simulink library "inp"  (Simulink 8.2)
settings: Solver=ode45  SolverMode=SingleTasking  StartTime=0.0  StopTime=10.0  RelTol=1e-3
undefined symbols (not defined in the file or in its folder): C_TICK_TIME_S, ENUM_FAULTSTATE_FAIL, ENUM_FAULTSTATE_INDETERMINATE, ENUM_FAULTSTATE_PASS, ENUM_KEYSWITCH_ON, FALSE, KINP_ABS1CANCommuOk_flg_ovrdflg, KINP_ABS1CANCommuOk_flg_ovrdval, KINP_ABS1CANPeriodTime_s, KINP_ABS1CANRolCntOk_flg_ovrdflg, KINP_ABS1CANRolCntOk_flg_ovrdval, KINP_ABS2CANChkSumOk_flg_ovrdflg, KINP_ABS2CANChkSumOk_flg_ovrdval, KINP_ABS2CANCommuOk_flg_ovrdflg, KINP_ABS2CANCommuOk_flg_ovrdval, KINP_ABS2CANPeriodTime_s, KINP_ABS2CANRolCntOk_flg_ovrdflg, KINP_ABS2CANRolCntOk_flg_ovrdval, KINP_ABS3CANCommuOk_flg_ovrdflg, KINP_ABS3CANCommuOk_flg_ovrdval, KINP_ABS4CANCommuOk_flg_ovrdflg, KINP_ABS4CANCommuOk_flg_ovrdval, KINP_ABSActive_flg_defval, KINP_ABSActive_flg_invalid, KINP_ABSActive_flg_ovrdflg, KINP_ABSActive_flg_ovrdval, KINP_ABSActive_flg_setvalid, KINP_ABSActive_flg_srvcflg, KINP_ABSActive_flg_srvcval, KINP_ABSCANAlive_flg_ovrdflg, KINP_ABSCANAlive_flg_ovrdval, KINP_ABSCANCommuOk_flg_ovrdflg, KINP_ABSCANCommuOk_flg_ovrdval, KINP_ABSCANValidOk_flg_ovrdflg, KINP_ABSCANValidOk_flg_ovrdval, KINP_ABSFault_flg_defval, KINP_ABSFault_flg_invalid, KINP_ABSFault_flg_ovrdflg, KINP_ABSFault_flg_ovrdval, KINP_ABSFault_flg_setvalid, KINP_ABSFault_flg_srvcflg, KINP_ABSFault_flg_srvcval, KINP_AC1CANCommuOk_flg_ovrdflg, KINP_AC1CANCommuOk_flg_ovrdval, KINP_ACCANAlive_flg_ovrdflg, KINP_ACCANAlive_flg_ovrdval, KINP_ACCANCommuOk_flg_ovrdflg, KINP_ACCANCommuOk_flg_ovrdval, KINP_ACCANValidOk_flg_ovrdflg, KINP_ACCANValidOk_flg_ovrdval, KINP_ACCCancelH_flg_defval, KINP_ACCCancelH_flg_invalid, KINP_ACCCancelH_flg_ovrdflg, KINP_ACCCancelH_flg_ovrdval, KINP_ACCCancelH_flg_setvalid, KINP_ACCCancelH_flg_srvcflg, KINP_ACCCancelH_flg_srvcval, KINP_AccelPedalPosn_pct_defval, KINP_AccelPedalPosn_pct_gain, KINP_AccelPedalPosn_pct_invalid ... (+1866)
libraries: inp x192, hcu_lib x34, simulink x33
(part 15 of 21)

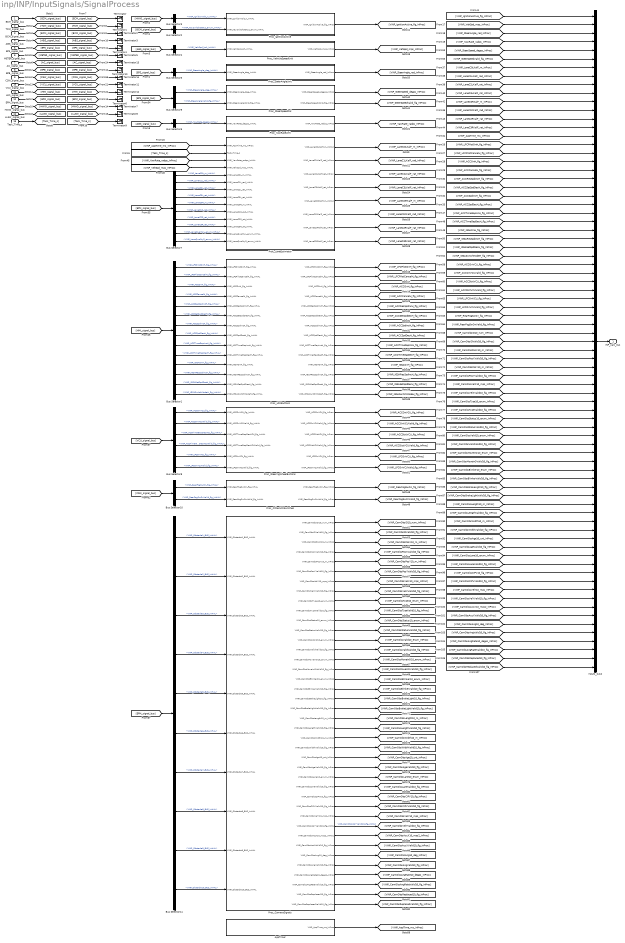
== system inp/INP/InputSignals/SignalProcess ==
  ECM_signal_bus : Inport
  TCM_signal_bus : Inport  Port=2
  BCM_signal_bus : Inport  Port=3
  ABS_signal_bus : Inport  Port=4
  EPS_signal_bus : Inport  Port=5
  METER_signal_bus : Inport  Port=6
  AC_signal_bus : Inport  Port=7
  EPB_signal_bus : Inport  Port=8
  CSW_signal_bus : Inport  Port=9
  VCU_signal_bus : Inport  Port=10
  HMI_signal_bus : Inport  Port=11
  EPM_signal_bus : Inport  Port=12
  HWIO_signal_bus : Inport  Port=13
  LLSW_signal_bus : Inport  Port=14
  Task_Time_s : Inport  Port=15
  AppTimer : Subsystem
  Bus Selector1 : BusSelector  OutputSignals=VINP_LFCPilotOnH_flg_InHAL,VINP_LFCPilotCancelH_flg_InHAL,VINP_ACCOnH_flg_InHAL,VINP_ACCCancelH_flg_InHAL,VINP_ACCResSpdIncH_flg_InHAL,VINP_ACCSetSpdDecH_flg_InHAL,VINP_ACCSpdIncH_flg_InHAL,VINP_ACCSpdDecH_flg_InHAL,VINP_ACCTimeGapIncH_flg_InHAL,VINP_ACCTimeGapDecH_flg_InHAL,VINP_IGSAOnH_flg_InHAL,VINP_IGSAResSpdIncH_flg_InHAL,VINP_IGSASetSpdDecH_flg_InHAL,VINP_IGSASwitchModeH_flg_InHAL
  Bus Selector10 : BusSelector  OutputSignals=VINP_RearFogSwOn_flg_InHAL,VINP_RearFogSwOnValid_flg_InHAL
  Bus Selector11 : BusSelector  OutputSignals=VINP_Obstacle1_BUS_InHAL,VINP_Obstacle2_BUS_InHAL,VINP_Obstacle3_BUS_InHAL,VINP_Obstacle4_BUS_InHAL,VINP_Obstacle5_BUS_InHAL,VINP_Obstacle6_BUS_InHAL,VINP_Obstacle7_BUS_InHAL,VINP_Obstacle8_BUS_InHAL,VINP_Obstacle9_BUS_InHAL,VINP_Obstacle10_BUS_InHAL
  Bus Selector2 : BusSelector  OutputSignals=VINP_KeySwitchStatus_enum_InHAL
  Bus Selector3 : BusSelector  OutputSignals=VINP_IgnitionVolt_V_InHAL
  Bus Selector4 : BusSelector  OutputSignals=VINP_VehSpd_kph_InHAL
  Bus Selector5 : BusSelector  OutputSignals=VINP_SteerAngle_deg_InHAL
  Bus Selector6 : BusSelector  OutputSignals=VINP_YawRate_degps_InHAL
  Bus Selector7 : BusSelector  OutputSignals=VINP_LaneC0L_m_InHAL,VINP_LaneC1L_rad_InHAL,VINP_LaneC2L_rat_InHAL,VINP_LaneC3L_rat_InHAL,VINP_LaneC0R_m_InHAL,VINP_LaneC1R_rad_InHAL,VINP_LaneC2R_rat_InHAL,VINP_LaneC3R_rat_InHAL,VINP_LaneQualityL_enum_InHAL,VINP_LaneQualityR_enum_InHAL
  Bus Selector8 : BusSelector  OutputSignals=VINP_ACCOnVCU_flg_InHAL,VINP_ACCOnVCUValid_flg_InHAL,VINP_ACCTimeGapDecVCU_flg_InHAL,VINP_ACCTimeGapDecVCUValid_flg_InHAL,VINP_LFCOnVCU_flg_InHAL,VINP_LFCOnVCUValid_flg_InHAL
  Bus Selector9 : BusSelector  OutputSignals=VINP_SteerAngle_deg_InHAL,VINP_SteerAngleValid_flg_InHAL
  Proc_CameraSignals : Subsystem
  Proc_HMIButtons : Subsystem
  Proc_IgnitionActive : Subsystem
  Proc_LaneEstimator : Subsystem
  Proc_OtherHMISwitches : Subsystem
  Proc_SteerAngleUnit : Subsystem
  Proc_SteerSpeedCal : Subsystem
  Proc_SteeringWheelButtons : Subsystem
  Proc_VehicleSpeedUnit : Subsystem
  Proc_YawRateUnit : Subsystem
  Terminator{,1..14} (15 alike) : Terminator
  inputs_bus2 : BusCreator  Inputs=77
  INP_calc_bus : Outport
  wires:
  [VINP_YawRate_radps_InProc] -> inputs_bus2/4
  [VINP_SteerAngle_rad_InProc] -> inputs_bus2/3
  [VINP_VehSpd_mps_InProc] -> inputs_bus2/2
  [VINP_IgnitionActive_flg_InProc] -> inputs_bus2/1
  AppTimer/1(VINP_AppTime_ms_InProc) -> [VINP_AppTime_ms_InProc]
  LLSW_signal_bus/1 -> [LLSW_signal_bus]
  Task_Time_s/1 -> [Task_Time_s]
  HWIO_signal_bus/1 -> [HWIO_signal_bus]
  EPM_signal_bus/1 -> [EPM_signal_bus]
  EPS_signal_bus/1 -> [EPS_signal_bus]
  ABS_signal_bus/1 -> [ABS_signal_bus]
  BCM_signal_bus/1 -> [BCM_signal_bus]
  TCM_signal_bus/1 -> [TCM_signal_bus]
  ECM_signal_bus/1 -> [ECM_signal_bus]
  [BCM_signal_bus] -> Bus Selector2/1
  [HWIO_signal_bus] -> Bus Selector3/1
  Bus Selector3/1 -> Proc_IgnitionActive/1(VINP_IgnitionVolt_V_InHAL)  "<VINP_IgnitionVolt_V_InHAL>"
  Bus Selector2/1 -> Proc_IgnitionActive/2(VINP_KeySwitchStatus_enum_InHAL)  "<VINP_KeySwitchStatus_enum_InHAL>"
  Proc_IgnitionActive/1(VINP_IgnitionActive_flg_InProc) -> [VINP_IgnitionActive_flg_InProc]
  [ABS_signal_bus] -> Bus Selector4/1
  Bus Selector4/1 -> Proc_VehicleSpeedUnit/1(VINP_VehSpd_kph_InHAL)  "<VINP_VehSpd_kph_InHAL>"
  Proc_VehicleSpeedUnit/1(VINP_VehSpd_mps_InProc) -> [VINP_VehSpd_mps_InProc]
  [EPS_signal_bus] -> Bus Selector5/1
  Bus Selector5/1 -> Proc_SteerAngleUnit/1(VINP_SteerAngle_deg_InHAL)  "<VINP_SteerAngle_deg_InHAL>"
  [ABS_signal_bus] -> Bus Selector6/1
  Bus Selector6/1 -> Proc_YawRateUnit/1(VINP_YawRate_degps_InHAL)  "<VINP_YawRate_degps_InHAL>"
  Proc_SteerAngleUnit/1(VINP_SteerAngle_rad_InProc) -> [VINP_SteerAngle_rad_InProc]
  Proc_YawRateUnit/1(VINP_YawRate_radps_InProc) -> [VINP_YawRate_radps_InProc]
  [ECM_signal_bus] -> Terminator/1
  [TCM_signal_bus] -> Terminator1/1
  [BCM_signal_bus] -> Terminator2/1
  [ABS_signal_bus] -> Terminator3/1
  [EPS_signal_bus] -> Terminator4/1
  [EPM_signal_bus] -> Terminator6/1
  [HWIO_signal_bus] -> Terminator7/1
  [LLSW_signal_bus] -> Terminator8/1
  [Task_Time_s] -> Terminator9/1
  inputs_bus2/1 -> INP_calc_bus/1
  [VINP_AppTime_ms_InProc] -> inputs_bus2/15
  [VINP_LaneC0LKalF_m_InProc] -> inputs_bus2/7
  [VINP_LaneC1LKalF_rad_InProc] -> inputs_bus2/8
  [VINP_LaneC2LKalF_rat_InProc] -> inputs_bus2/9
  [VINP_LaneC3LKalF_rat_InProc] -> inputs_bus2/10
  [VINP_LaneC0RKalF_m_InProc] -> inputs_bus2/11
  [VINP_LaneC1RKalF_rad_InProc] -> inputs_bus2/12
  [VINP_LaneC2RKalF_rat_InProc] -> inputs_bus2/13
  [VINP_LaneC3RKalF_rat_InProc] -> inputs_bus2/14
  [EPM_signal_bus] -> Bus Selector7/1
  Bus Selector7/1 -> Proc_LaneEstimator/5(VINP_LaneC0L_m_InHAL)  "<VINP_LaneC0L_m_InHAL>"
  Bus Selector7/2 -> Proc_LaneEstimator/6(VINP_LaneC1L_rad_InHAL)  "<VINP_LaneC1L_rad_InHAL>"
  Bus Selector7/3 -> Proc_LaneEstimator/7(VINP_LaneC2L_rat_InHAL)  "<VINP_LaneC2L_rat_InHAL>"
  Bus Selector7/4 -> Proc_LaneEstimator/8(VINP_LaneC3L_rat_InHAL)  "<VINP_LaneC3L_rat_InHAL>"
  Bus Selector7/5 -> Proc_LaneEstimator/9(VINP_LaneC0R_m_InHAL)  "<VINP_LaneC0R_m_InHAL>"
  Bus Selector7/6 -> Proc_LaneEstimator/10(VINP_LaneC1R_rad_InHAL)  "<VINP_LaneC1R_rad_InHAL>"
  Bus Selector7/7 -> Proc_LaneEstimator/11(VINP_LaneC2R_rat_InHAL)  "<VINP_LaneC2R_rat_InHAL>"
  Bus Selector7/8 -> Proc_LaneEstimator/12(VINP_LaneC3R_rat_InHAL)  "<VINP_LaneC3R_rat_InHAL>"
  [VINP_YawRate_radps_InProc] -> Proc_LaneEstimator/3(VINP_YawRate_radps_InHAL)
  [VINP_VehSpd_mps_InProc] -> Proc_LaneEstimator/4(VINP_VehSpd_mps_InHAL)
  Bus Selector7/9 -> Proc_LaneEstimator/13(VINP_LaneQualityL_enum_InHAL)  "<VINP_LaneQualityL_enum_InHAL>"
  Bus Selector7/10 -> Proc_LaneEstimator/14(VINP_LaneQualityR_enum_InHAL)  "<VINP_LaneQualityR_enum_InHAL>"
  [Task_Time_s] -> Proc_LaneEstimator/2(Task_Time_s)
  [VINP_AppTime_ms_InProc] -> Proc_LaneEstimator/1(VINP_AppTime_ms_InProc)
  Proc_LaneEstimator/1(VINP_LaneC0LKalF_m_InProc) -> [VINP_LaneC0LKalF_m_InProc]
  Proc_LaneEstimator/2(VINP_LaneC1LKalF_rad_InProc) -> [VINP_LaneC1LKalF_rad_InProc]
  Proc_LaneEstimator/3(VINP_LaneC2LKalF_rat_InProc) -> [VINP_LaneC2LKalF_rat_InProc]
  Proc_LaneEstimator/4(VINP_LaneC3LKalF_rat_InProc) -> [VINP_LaneC3LKalF_rat_InProc]
  Proc_LaneEstimator/5(VINP_LaneC0RKalF_m_InProc) -> [VINP_LaneC0RKalF_m_InProc]
  Proc_LaneEstimator/6(VINP_LaneC1RKalF_rad_InProc) -> [VINP_LaneC1RKalF_rad_InProc]
  Proc_LaneEstimator/7(VINP_LaneC2RKalF_rat_InProc) -> [VINP_LaneC2RKalF_rat_InProc]
  Proc_LaneEstimator/8(VINP_LaneC3RKalF_rat_InProc) -> [VINP_LaneC3RKalF_rat_InProc]
  [METER_signal_bus] -> Terminator5/1
  METER_signal_bus/1 -> [METER_signal_bus]
  [AC_signal_bus] -> Terminator13/1
  AC_signal_bus/1 -> [AC_signal_bus]
  [EPB_signal_bus] -> Terminator10/1
  EPB_signal_bus/1 -> [EPB_signal_bus]
  [VCU_signal_bus] -> Terminator11/1
  VCU_signal_bus/1 -> [VCU_signal_bus]
  [HMI_signal_bus] -> Terminator12/1
  HMI_signal_bus/1 -> [HMI_signal_bus]
  [HMI_signal_bus] -> Bus Selector1/1
  Bus Selector1/1 -> Proc_HMIButtons/1(VINP_LFCPilotOnH_flg_InHAL)  "<VINP_LFCPilotOnH_flg_InHAL>"
  Proc_HMIButtons/1(VINP_LFCPilotOnH_flg_InProc) -> [VINP_LFCPilotOnH_flg_InProc]
  Bus Selector1/2 -> Proc_HMIButtons/2(VINP_LFCPilotCancelH_flg_InHAL)  "<VINP_LFCPilotCancelH_flg_InHAL>"
  Bus Selector1/3 -> Proc_HMIButtons/3(VINP_ACCOnH_flg_InHAL)  "<VINP_ACCOnH_flg_InHAL>"
  Bus Selector1/4 -> Proc_HMIButtons/4(VINP_ACCCancelH_flg_InHAL)  "<VINP_ACCCancelH_flg_InHAL>"
  Bus Selector1/5 -> Proc_HMIButtons/5(VINP_ACCResSpdIncH_flg_InHAL)  "<VINP_ACCResSpdIncH_flg_InHAL>"
  Bus Selector1/6 -> Proc_HMIButtons/6(VINP_ACCSetSpdDecH_flg_InHAL)  "<VINP_ACCSetSpdDecH_flg_InHAL>"
  Bus Selector1/7 -> Proc_HMIButtons/7(VINP_ACCSpdIncH_flg_InHAL)  "<VINP_ACCSpdIncH_flg_InHAL>"
  Bus Selector1/8 -> Proc_HMIButtons/8(VINP_ACCSpdDecH_flg_InHAL)  "<VINP_ACCSpdDecH_flg_InHAL>"
  Bus Selector1/9 -> Proc_HMIButtons/9(VINP_ACCTimeGapIncH_flg_InHAL)  "<VINP_ACCTimeGapIncH_flg_InHAL>"
  Bus Selector1/10 -> Proc_HMIButtons/10(VINP_ACCTimeGapDecH_flg_InHAL)  "<VINP_ACCTimeGapDecH_flg_InHAL>"
  Bus Selector1/11 -> Proc_HMIButtons/11(VINP_IGSAOnH_flg_InHAL)  "<VINP_IGSAOnH_flg_InHAL>"
  Bus Selector1/12 -> Proc_HMIButtons/12(VINP_IGSAResSpdIncH_flg_InHAL)  "<VINP_IGSAResSpdIncH_flg_InHAL>"
  Bus Selector1/13 -> Proc_HMIButtons/13(VINP_IGSASetSpdDecH_flg_InHAL)  "<VINP_IGSASetSpdDecH_flg_InHAL>"
  Bus Selector1/14 -> Proc_HMIButtons/14(VINP_IGSASwitchModeH_flg_InHAL)  "<VINP_IGSASwitchModeH_flg_InHAL>"
  Proc_HMIButtons/2(VINP_LFCPilotCancelH_flg_InProc) -> [VINP_LFCPilotCancelH_flg_InProc]
  Proc_HMIButtons/3(VINP_ACCOnH_flg_InProc) -> [VINP_ACCOnH_flg_InProc]
  Proc_HMIButtons/4(VINP_ACCCancelH_flg_InProc) -> [VINP_ACCCancelH_flg_InProc]
  Proc_HMIButtons/5(VINP_ACCResSpdIncH_flg_InProc) -> [VINP_ACCResSpdIncH_flg_InProc]
  Proc_HMIButtons/6(VINP_ACCSetSpdDecH_flg_InProc) -> [VINP_ACCSetSpdDecH_flg_InProc]
  Proc_HMIButtons/7(VINP_ACCSpdIncH_flg_InProc) -> [VINP_ACCSpdIncH_flg_InProc]
  Proc_HMIButtons/8(VINP_ACCSpdDecH_flg_InProc) -> [VINP_ACCSpdDecH_flg_InProc]
  Proc_HMIButtons/9(VINP_ACCTimeGapIncH_flg_InProc) -> [VINP_ACCTimeGapIncH_flg_InProc]
  Proc_HMIButtons/10(VINP_ACCTimeGapDecH_flg_InProc) -> [VINP_ACCTimeGapDecH_flg_InProc]
  Proc_HMIButtons/11(VINP_IGSAOnH_flg_InProc) -> [VINP_IGSAOnH_flg_InProc]
  Proc_HMIButtons/12(VINP_IGSAResSpdIncH_flg_InProc) -> [VINP_IGSAResSpdIncH_flg_InProc]
  Proc_HMIButtons/13(VINP_IGSASetSpdDecH_flg_InProc) -> [VINP_IGSASetSpdDecH_flg_InProc]
  Proc_HMIButtons/14(VINP_IGSASwitchModeH_flg_InProc) -> [VINP_IGSASwitchModeH_flg_InProc]
  [VINP_LFCPilotOnH_flg_InProc] -> inputs_bus2/16
  [VINP_LFCPilotCancelH_flg_InProc] -> inputs_bus2/17
  [VINP_ACCOnH_flg_InProc] -> inputs_bus2/18
  [VINP_ACCCancelH_flg_InProc] -> inputs_bus2/19
  [VINP_ACCResSpdIncH_flg_InProc] -> inputs_bus2/20
  [VINP_ACCSetSpdDecH_flg_InProc] -> inputs_bus2/21
  [VINP_ACCSpdIncH_flg_InProc] -> inputs_bus2/22
  [VINP_ACCSpdDecH_flg_InProc] -> inputs_bus2/23
  [VINP_ACCTimeGapIncH_flg_InProc] -> inputs_bus2/24
  [VINP_ACCTimeGapDecH_flg_InProc] -> inputs_bus2/25
  [VINP_IGSAOnH_flg_InProc] -> inputs_bus2/26
  [VINP_IGSAResSpdIncH_flg_InProc] -> inputs_bus2/27
  [VINP_IGSASetSpdDecH_flg_InProc] -> inputs_bus2/28
  [VINP_IGSASwitchModeH_flg_InProc] -> inputs_bus2/29
  Proc_SteerSpeedCal/1(VINP_SteerSpeed_degps_InProc) -> [VINP_SteerSpeed_degps_InProc]
  [EPS_signal_bus] -> Bus Selector9/1
  Bus Selector9/1 -> Proc_SteerSpeedCal/1(VINP_SteerAngle_deg_InHAL)  "<VINP_SteerAngle_deg_InHAL>"
  Bus Selector9/2 -> Proc_SteerSpeedCal/2(VINP_SteerAngleValid_flg_InHAL)  "<VINP_SteerAngleValid_flg_InHAL>"
  Proc_SteerSpeedCal/2(VINP_SteerSpeedValid_flg_InProc) -> [VINP_SteerSpeedValid_flg_InProc]
  [VINP_SteerSpeed_degps_InProc] -> inputs_bus2/5
  [VINP_SteerSpeedValid_flg_InProc] -> inputs_bus2/6
  [CSW_signal_bus] -> Terminator14/1
  CSW_signal_bus/1 -> [CSW_signal_bus]
  [VCU_signal_bus] -> Bus Selector8/1
  Bus Selector8/2 -> Proc_SteeringWheelButtons/2(VINP_ACCOnVCUValid_flg_InHAL)  "<VINP_ACCOnVCUValid_flg_InHAL>"
  Bus Selector8/3 -> Proc_SteeringWheelButtons/3(VINP_ACCTimeGapDecVCU_flg_InHAL)  "<VINP_ACCTimeGapDecVCU_flg_InHAL>"
  Bus Selector8/1 -> Proc_SteeringWheelButtons/1(VINP_ACCOnVCU_flg_InHAL)  "<VINP_ACCOnVCU_flg_InHAL>"
  Bus Selector8/4 -> Proc_SteeringWheelButtons/4(VINP_ACCTimeGapDecVCUValid_flg_InHAL)  "<VINP_ACCTimeGapDecVCUValid_flg_InHAL>"
  Proc_SteeringWheelButtons/1(VINP_ACCOnVCU_flg_InProc) -> [VINP_ACCOnVCU_flg_InProc]
  Proc_SteeringWheelButtons/2(VINP_ACCOnVCUValid_flg_InProc) -> [VINP_ACCOnVCUValid_flg_InProc]
  Proc_SteeringWheelButtons/3(VINP_ACCDistVCU_flg_InProc) -> [VINP_ACCDistVCU_flg_InProc]
  Proc_SteeringWheelButtons/4(VINP_ACCDistVCUValid_flg_InProc) -> [VINP_ACCDistVCUValid_flg_InProc]
  [VINP_ACCOnVCU_flg_InProc] -> inputs_bus2/30
  [VINP_ACCOnVCUValid_flg_InProc] -> inputs_bus2/31
  [VINP_ACCDistVCU_flg_InProc] -> inputs_bus2/32
  [VINP_ACCDistVCUValid_flg_InProc] -> inputs_bus2/33
  [CSW_signal_bus] -> Bus Selector10/1
  Bus Selector10/1 -> Proc_OtherHMISwitches/1(VINP_RearFogSwOn_flg_InHAL)  "<VINP_RearFogSwOn_flg_InHAL>"
  Proc_OtherHMISwitches/1(VINP_RearFogSwOn_flg_InProc) -> [VINP_RearFogSwOn_flg_InProc]
  [VINP_RearFogSwOn_flg_InProc] -> inputs_bus2/36
  Bus Selector10/2 -> Proc_OtherHMISwitches/2(VINP_RearFogSwOnValid_flg_InHAL)  "<VINP_RearFogSwOnValid_flg_InHAL>"
  Proc_OtherHMISwitches/2(VINP_RearFogSwOnValid_flg_InProc) -> [VINP_RearFogSwOnValid_flg_InProc]
  [VINP_RearFogSwOnValid_flg_InProc] -> inputs_bus2/37
  Bus Selector8/5 -> Proc_SteeringWheelButtons/5(VINP_LFCOnVCU_flg_InHAL)  "<VINP_LFCOnVCU_flg_InHAL>"
  Bus Selector8/6 -> Proc_SteeringWheelButtons/6(VINP_LFCOnVCUValid_flg_InHAL)  "<VINP_LFCOnVCUValid_flg_InHAL>"
  Proc_SteeringWheelButtons/5(VINP_LFCOnVCU_flg_InProc) -> [VINP_LFCOnVCU_flg_InProc]
  Proc_SteeringWheelButtons/6(VINP_LFCOnVCUValid_flg_InProc) -> [VINP_LFCOnVCUValid_flg_InProc]
  [VINP_LFCOnVCU_flg_InProc] -> inputs_bus2/34
  [VINP_LFCOnVCUValid_flg_InProc] -> inputs_bus2/35
  [EPM_signal_bus] -> Bus Selector11/1
  Bus Selector11/1 -> Proc_CameraSignals/1(VINP_Obstacle1_BUS_InHAL)  "<VINP_Obstacle1_BUS_InHAL>"
  Bus Selector11/2 -> Proc_CameraSignals/2(VINP_Obstacle2_BUS_InHAL)  "<VINP_Obstacle2_BUS_InHAL>"
  Bus Selector11/3 -> Proc_CameraSignals/3(VINP_Obstacle3_BUS_InHAL)  "<VINP_Obstacle3_BUS_InHAL>"
  Bus Selector11/4 -> Proc_CameraSignals/4(VINP_Obstacle4_BUS_InHAL)  "<VINP_Obstacle4_BUS_InHAL>"
  Bus Selector11/5 -> Proc_CameraSignals/5(VINP_Obstacle5_BUS_InHAL)  "<VINP_Obstacle5_BUS_InHAL>"
  Bus Selector11/6 -> Proc_CameraSignals/6(VINP_Obstacle6_BUS_InHAL)  "<VINP_Obstacle6_BUS_InHAL>"
  Bus Selector11/7 -> Proc_CameraSignals/7(VINP_Obstacle7_BUS_InHAL)  "<VINP_Obstacle7_BUS_InHAL>"
  Bus Selector11/8 -> Proc_CameraSignals/8(VINP_Obstacle8_BUS_InHAL)  "<VINP_Obstacle8_BUS_InHAL>"
  Bus Selector11/9 -> Proc_CameraSignals/9(VINP_Obstacle9_BUS_InHAL)  "<VINP_Obstacle9_BUS_InHAL>"
  Bus Selector11/10 -> Proc_CameraSignals/10(VINP_Obstacle10_BUS_InHAL)  "<VINP_Obstacle10_BUS_InHAL>"
  Proc_CameraSignals/1(VINP_CamObsID10_num_InProc) -> [VINP_CamObsID10_num_InProc]
  Proc_CameraSignals/2(VINP_CamObsIDValid10_flg_InProc) -> [VINP_CamObsIDValid10_flg_InProc]
  Proc_CameraSignals/3(VINP_CamObsPosX10_m_InProc) -> [VINP_CamObsPosX10_m_InProc]
  Proc_CameraSignals/4(VINP_CamObsPosXValid10_flg_InProc) -> [VINP_CamObsPosXValid10_flg_InProc]
  Proc_CameraSignals/5(VINP_CamObsPosY10_m_InProc) -> [VINP_CamObsPosY10_m_InProc]
  Proc_CameraSignals/6(VINP_CamObsPosYValid10_flg_InProc) -> [VINP_CamObsPosYValid10_flg_InProc]
  Proc_CameraSignals/7(VINP_CamObsVelX10_mps_InProc) -> [VINP_CamObsVelX10_mps_InProc]
  Proc_CameraSignals/8(VINP_CamObsVelXValid10_flg_InProc) -> [VINP_CamObsVelXValid10_flg_InProc]
  Proc_CameraSignals/9(VINP_CamObsType10_enum_InProc) -> [VINP_CamObsType10_enum_InProc]
  Proc_CameraSignals/10(VINP_CamObsTypeValid10_flg_InProc) -> [VINP_CamObsTypeValid10_flg_InProc]
  Proc_CameraSignals/11(VINP_CamObsStatus10_enum_InProc) -> [VINP_CamObsStatus10_enum_InProc]
  Proc_CameraSignals/12(VINP_CamObsStatusValid10_flg_InProc) -> [VINP_CamObsStatusValid10_flg_InProc]
  Proc_CameraSignals/13(VINP_CamObsValid10_enum_InProc) -> [VINP_CamObsValid10_enum_InProc]
  Proc_CameraSignals/14(VINP_CamObsValidValid10_flg_InProc) -> [VINP_CamObsValidValid10_flg_InProc]
  Proc_CameraSignals/15(VINP_CamObsMoveInO10_enum_InProc) -> [VINP_CamObsMoveInO10_enum_InProc]
  Proc_CameraSignals/16(VINP_CamObsMoveInOValid10_flg_InProc) -> [VINP_CamObsMoveInOValid10_flg_InProc]
  Proc_CameraSignals/17(VINP_CamObsBlinker10_enum_InProc) -> [VINP_CamObsBlinker10_enum_InProc]
  Proc_CameraSignals/18(VINP_CamObsBlinkerValid10_flg_InProc) -> [VINP_CamObsBlinkerValid10_flg_InProc]
  Proc_CameraSignals/19(VINP_CamObsBrakeLight10_flg_InProc) -> [VINP_CamObsBrakeLight10_flg_InProc]
  Proc_CameraSignals/20(VINP_CamObsBrakeLightValid10_flg_InProc) -> [VINP_CamObsBrakeLightValid10_flg_InProc]
  Proc_CameraSignals/21(VINP_CamObsLength10_m_InProc) -> [VINP_CamObsLength10_m_InProc]
  Proc_CameraSignals/22(VINP_CamObsLengthValid10_flg_InProc) -> [VINP_CamObsLengthValid10_flg_InProc]
  Proc_CameraSignals/23(VINP_CamObsWidth10_m_InProc) -> [VINP_CamObsWidth10_m_InProc]
  Proc_CameraSignals/24(VINP_CamObsWidthValid10_flg_InProc) -> [VINP_CamObsWidthValid10_flg_InProc]
  Proc_CameraSignals/25(VINP_CamObsAge10_cnt_InProc) -> [VINP_CamObsAge10_cnt_InProc]
  Proc_CameraSignals/26(VINP_CamObsAgeValid10_flg_InProc) -> [VINP_CamObsAgeValid10_flg_InProc]
  Proc_CameraSignals/27(VINP_CamObsLane10_enum_InProc) -> [VINP_CamObsLane10_enum_InProc]
  Proc_CameraSignals/28(VINP_CamObsLaneValid10_flg_InProc) -> [VINP_CamObsLaneValid10_flg_InProc]
  Proc_CameraSignals/29(VINP_CamObsCIPV10_flg_InProc) -> [VINP_CamObsCIPV10_flg_InProc]
  Proc_CameraSignals/30(VINP_CamObsCIPVValid10_flg_InProc) -> [VINP_CamObsCIPVValid10_flg_InProc]
  Proc_CameraSignals/31(VINP_CamObsVelY10_mps_InProc) -> [VINP_CamObsVelY10_mps_InProc]
  Proc_CameraSignals/32(VINP_CamObsVelYValid10_flg_InProc) -> [VINP_CamObsVelYValid10_flg_InProc]  "VINP_CamObsVelYValid10_flg_InProc"
  Proc_CameraSignals/33(VINP_CamObsAccX10_mps2_InProc) -> [VINP_CamObsAccX10_mps2_InProc]
  Proc_CameraSignals/34(VINP_CamObsAccXValid10_flg_InProc) -> [VINP_CamObsAccXValid10_flg_InProc]
  Proc_CameraSignals/35(VINP_CamObsAng10_deg_InProc) -> [VINP_CamObsAng10_deg_InProc]
  Proc_CameraSignals/36(VINP_CamObsAngValid10_flg_InProc) -> [VINP_CamObsAngValid10_flg_InProc]
  Proc_CameraSignals/37(VINP_CamObsAngRate10_degps_InProc) -> [VINP_CamObsAngRate10_degps_InProc]
  Proc_CameraSignals/38(VINP_CamObsAngRateValid10_flg_InProc) -> [VINP_CamObsAngRateValid10_flg_InProc]
  Proc_CameraSignals/39(VINP_CamObsReplaced10_flg_InProc) -> [VINP_CamObsReplaced10_flg_InProc]
  Proc_CameraSignals/40(VINP_CamObsReplacedValid10_flg_InProc) -> [VINP_CamObsReplacedValid10_flg_InProc]
  [VINP_CamObsID10_num_InProc] -> inputs_bus2/38
  [VINP_CamObsIDValid10_flg_InProc] -> inputs_bus2/39
  [VINP_CamObsPosX10_m_InProc] -> inputs_bus2/40
  [VINP_CamObsPosXValid10_flg_InProc] -> inputs_bus2/41
  [VINP_CamObsPosY10_m_InProc] -> inputs_bus2/42
  [VINP_CamObsPosYValid10_flg_InProc] -> inputs_bus2/43
  [VINP_CamObsVelX10_mps_InProc] -> inputs_bus2/44
  [VINP_CamObsVelXValid10_flg_InProc] -> inputs_bus2/45
  [VINP_CamObsType10_enum_InProc] -> inputs_bus2/46
  [VINP_CamObsTypeValid10_flg_InProc] -> inputs_bus2/47
  [VINP_CamObsStatus10_enum_InProc] -> inputs_bus2/48
  [VINP_CamObsStatusValid10_flg_InProc] -> inputs_bus2/49
  [VINP_CamObsValid10_enum_InProc] -> inputs_bus2/50
  [VINP_CamObsValidValid10_flg_InProc] -> inputs_bus2/51
  [VINP_CamObsMoveInO10_enum_InProc] -> inputs_bus2/52
  [VINP_CamObsMoveInOValid10_flg_InProc] -> inputs_bus2/53
  [VINP_CamObsBlinker10_enum_InProc] -> inputs_bus2/54
  [VINP_CamObsBlinkerValid10_flg_InProc] -> inputs_bus2/55
  [VINP_CamObsBrakeLight10_flg_InProc] -> inputs_bus2/56
  [VINP_CamObsBrakeLightValid10_flg_InProc] -> inputs_bus2/57
  [VINP_CamObsLength10_m_InProc] -> inputs_bus2/58
  [VINP_CamObsLengthValid10_flg_InProc] -> inputs_bus2/59
  [VINP_CamObsWidth10_m_InProc] -> inputs_bus2/60
  [VINP_CamObsWidthValid10_flg_InProc] -> inputs_bus2/61
  [VINP_CamObsAge10_cnt_InProc] -> inputs_bus2/62
  [VINP_CamObsAgeValid10_flg_InProc] -> inputs_bus2/63
  [VINP_CamObsLane10_enum_InProc] -> inputs_bus2/64
  [VINP_CamObsLaneValid10_flg_InProc] -> inputs_bus2/65
  [VINP_CamObsCIPV10_flg_InProc] -> inputs_bus2/66
  [VINP_CamObsCIPVValid10_flg_InProc] -> inputs_bus2/67
  [VINP_CamObsVelY10_mps_InProc] -> inputs_bus2/68
  [VINP_CamObsVelYValid10_flg_InProc] -> inputs_bus2/69
  [VINP_CamObsAccX10_mps2_InProc] -> inputs_bus2/70
  [VINP_CamObsAccXValid10_flg_InProc] -> inputs_bus2/71
  [VINP_CamObsAng10_deg_InProc] -> inputs_bus2/72
  [VINP_CamObsAngValid10_flg_InProc] -> inputs_bus2/73
  [VINP_CamObsAngRate10_degps_InProc] -> inputs_bus2/74
  [VINP_CamObsAngRateValid10_flg_InProc] -> inputs_bus2/75
  [VINP_CamObsReplaced10_flg_InProc] -> inputs_bus2/76
  [VINP_CamObsReplacedValid10_flg_InProc] -> inputs_bus2/77

== system inp/INP/METER_signals/LowBrakeFluid ==
  signal_in : Inport
  msg_valid : Inport  Port=2
  Active_fault_flg : Inport  Port=3
  can_signal_process_wio_validity : inp/can_signal_process_wio_validity
  defval : Constant  Value=KINP_LowBrakeFluid_flg_defval
  invalid : Constant  Value=KINP_LowBrakeFluid_flg_invalid
  ovrdflg : Constant  Value=KINP_LowBrakeFluid_flg_ovrdflg
  ovrdval : Constant  Value=KINP_LowBrakeFluid_flg_ovrdval
  setvalid : Constant  Value=KINP_LowBrakeFluid_flg_setvalid
  srvcflg : Constant  Value=KINP_LowBrakeFluid_flg_srvcflg
  srvcval : Constant  Value=KINP_LowBrakeFluid_flg_srvcval
  signal_out : Outport
  signal_valid_out : Outport  Port=2
  wires:
  can_signal_process_wio_validity/1 -> signal_out/1
  can_signal_process_wio_validity/2 -> signal_valid_out/1
  signal_in/1 -> can_signal_process_wio_validity/1
  msg_valid/1 -> can_signal_process_wio_validity/2
  defval/1 -> can_signal_process_wio_validity/4
  invalid/1 -> can_signal_process_wio_validity/5
  setvalid/1 -> can_signal_process_wio_validity/6
  ovrdflg/1 -> can_signal_process_wio_validity/7
  ovrdval/1 -> can_signal_process_wio_validity/8
  srvcflg/1 -> can_signal_process_wio_validity/9
  srvcval/1 -> can_signal_process_wio_validity/10
  Active_fault_flg/1 -> can_signal_process_wio_validity/3

== system inp/INP/METER_signals/MeterVehSpd ==
  signal_in : Inport
  signal_valid_in : Inport  Port=2
  msg_valid : Inport  Port=3
  Active_fault_flg : Inport  Port=4
  can_signal_process_with_validity : inp/can_signal_process_with_validity
  conv_gain_offset : Subsystem
  defval : Constant  Value=KINP_MeterVehSpd_kph_defval
  invalid : Constant  Value=KINP_MeterVehSpd_kph_invalid
  min_max_limit : Subsystem
  ovrdflg : Constant  Value=KINP_MeterVehSpd_kph_ovrdflg
  ovrdval : Constant  Value=KINP_MeterVehSpd_kph_ovrdval
  setvalid : Constant  Value=KINP_MeterVehSpd_kph_setvalid
  srvcflg : Constant  Value=KINP_MeterVehSpd_kph_srvcflg
  srvcval : Constant  Value=KINP_MeterVehSpd_kph_srvcval
  signal_out : Outport
  signal_valid_out : Outport  Port=2
  raw_signal_valid_out : Outport  Port=3
  wires:
  can_signal_process_with_validity/1 -> min_max_limit/1(in)
  can_signal_process_with_validity/2 -> signal_valid_out/1
  signal_in/1 -> conv_gain_offset/1(in)
  signal_valid_in/1 -> can_signal_process_with_validity/2
  msg_valid/1 -> can_signal_process_with_validity/3, can_signal_process_with_validity/5
  defval/1 -> can_signal_process_with_validity/6
  invalid/1 -> can_signal_process_with_validity/7
  conv_gain_offset/1(out) -> can_signal_process_with_validity/1
  min_max_limit/1(out) -> signal_out/1
  setvalid/1 -> can_signal_process_with_validity/8
  ovrdflg/1 -> can_signal_process_with_validity/9
  ovrdval/1 -> can_signal_process_with_validity/10
  srvcflg/1 -> can_signal_process_with_validity/11
  srvcval/1 -> can_signal_process_with_validity/12
  Active_fault_flg/1 -> can_signal_process_with_validity/4
  can_signal_process_with_validity/3 -> raw_signal_valid_out/1

== system inp/INP/METER_signals/OdoMeter ==
  signal_in : Inport
  signal_valid_in : Inport  Port=2
  msg_valid : Inport  Port=3
  Active_fault_flg : Inport  Port=4
  can_signal_process_with_validity : inp/can_signal_process_with_validity
  conv_gain_offset : Subsystem
  defval : Constant  Value=KINP_OdoMeter_km_defval
  invalid : Constant  Value=KINP_OdoMeter_km_invalid
  min_max_limit : Subsystem
  ovrdflg : Constant  Value=KINP_OdoMeter_km_ovrdflg
  ovrdval : Constant  Value=KINP_OdoMeter_km_ovrdval
  setvalid : Constant  Value=KINP_OdoMeter_km_setvalid
  srvcflg : Constant  Value=KINP_OdoMeter_km_srvcflg
  srvcval : Constant  Value=KINP_OdoMeter_km_srvcval
  signal_out : Outport
  signal_valid_out : Outport  Port=2
  raw_signal_valid_out : Outport  Port=3
  wires:
  can_signal_process_with_validity/3 -> raw_signal_valid_out/1
  Active_fault_flg/1 -> can_signal_process_with_validity/4
  srvcval/1 -> can_signal_process_with_validity/12
  srvcflg/1 -> can_signal_process_with_validity/11
  ovrdval/1 -> can_signal_process_with_validity/10
  ovrdflg/1 -> can_signal_process_with_validity/9
  setvalid/1 -> can_signal_process_with_validity/8
  min_max_limit/1(out) -> signal_out/1
  conv_gain_offset/1(out) -> can_signal_process_with_validity/1
  invalid/1 -> can_signal_process_with_validity/7
  defval/1 -> can_signal_process_with_validity/6
  msg_valid/1 -> can_signal_process_with_validity/5, can_signal_process_with_validity/3
  signal_valid_in/1 -> can_signal_process_with_validity/2
  signal_in/1 -> conv_gain_offset/1(in)
  can_signal_process_with_validity/2 -> signal_valid_out/1
  can_signal_process_with_validity/1 -> min_max_limit/1(in)

== system inp/INP/METER_signals/ParkBrakeOn ==
  signal_in : Inport
  msg_valid : Inport  Port=2
  Active_fault_flg : Inport  Port=3
  can_signal_process_wio_validity : inp/can_signal_process_wio_validity
  defval : Constant  Value=KINP_ParkBrakeOn_flg_defval
  invalid : Constant  Value=KINP_ParkBrakeOn_flg_invalid
  ovrdflg : Constant  Value=KINP_ParkBrakeOn_flg_ovrdflg
  ovrdval : Constant  Value=KINP_ParkBrakeOn_flg_ovrdval
  setvalid : Constant  Value=KINP_ParkBrakeOn_flg_setvalid
  srvcflg : Constant  Value=KINP_ParkBrakeOn_flg_srvcflg
  srvcval : Constant  Value=KINP_ParkBrakeOn_flg_srvcval
  signal_out : Outport
  signal_valid_out : Outport  Port=2
  wires:
  Active_fault_flg/1 -> can_signal_process_wio_validity/3
  srvcval/1 -> can_signal_process_wio_validity/10
  srvcflg/1 -> can_signal_process_wio_validity/9
  ovrdval/1 -> can_signal_process_wio_validity/8
  ovrdflg/1 -> can_signal_process_wio_validity/7
  setvalid/1 -> can_signal_process_wio_validity/6
  invalid/1 -> can_signal_process_wio_validity/5
  defval/1 -> can_signal_process_wio_validity/4
  msg_valid/1 -> can_signal_process_wio_validity/2
  signal_in/1 -> can_signal_process_wio_validity/1
  can_signal_process_wio_validity/2 -> signal_valid_out/1
  can_signal_process_wio_validity/1 -> signal_out/1

== system inp/INP/METER_signals/SeatBeltNOTFasten ==
  signal_in : Inport
  msg_valid : Inport  Port=2
  Active_fault_flg : Inport  Port=3
  can_signal_process_wio_validity : inp/can_signal_process_wio_validity
  defval : Constant  Value=KINP_SeatBeltNOTFasten_flg_defval
  invalid : Constant  Value=KINP_SeatBeltNOTFasten_flg_invalid
  ovrdflg : Constant  Value=KINP_SeatBeltNOTFasten_flg_ovrdflg
  ovrdval : Constant  Value=KINP_SeatBeltNOTFasten_flg_ovrdval
  setvalid : Constant  Value=KINP_SeatBeltNOTFasten_flg_setvalid
  srvcflg : Constant  Value=KINP_SeatBeltNOTFasten_flg_srvcflg
  srvcval : Constant  Value=KINP_SeatBeltNOTFasten_flg_srvcval
  signal_out : Outport
  signal_valid_out : Outport  Port=2
  wires:
  can_signal_process_wio_validity/1 -> signal_out/1
  can_signal_process_wio_validity/2 -> signal_valid_out/1
  signal_in/1 -> can_signal_process_wio_validity/1
  msg_valid/1 -> can_signal_process_wio_validity/2
  defval/1 -> can_signal_process_wio_validity/4
  invalid/1 -> can_signal_process_wio_validity/5
  setvalid/1 -> can_signal_process_wio_validity/6
  ovrdflg/1 -> can_signal_process_wio_validity/7
  ovrdval/1 -> can_signal_process_wio_validity/8
  srvcflg/1 -> can_signal_process_wio_validity/9
  srvcval/1 -> can_signal_process_wio_validity/10
  Active_fault_flg/1 -> can_signal_process_wio_validity/3

== system inp/INP/TCM_signals/DriverMode ==
  signal_in : Inport
  msg_valid : Inport  Port=2
  Active_fault_flg : Inport  Port=3
  can_signal_process_wio_validity : inp/can_signal_process_wio_validity
  defval : Constant  Value=KINP_DriverMode_enum_defval
  invalid : Constant  Value=KINP_DriverMode_enum_invalid
  ovrdflg : Constant  Value=KINP_DriverMode_enum_ovrdflg
  ovrdval : Constant  Value=KINP_DriverMode_enum_ovrdval
  setvalid : Constant  Value=KINP_DriverMode_enum_setvalid
  srvcflg : Constant  Value=KINP_DriverMode_enum_srvcflg
  srvcval : Constant  Value=KINP_DriverMode_enum_srvcval
  signal_out : Outport
  signal_valid_out : Outport  Port=2
  wires:
  can_signal_process_wio_validity/1 -> signal_out/1
  can_signal_process_wio_validity/2 -> signal_valid_out/1
  signal_in/1 -> can_signal_process_wio_validity/1
  msg_valid/1 -> can_signal_process_wio_validity/2
  defval/1 -> can_signal_process_wio_validity/4
  invalid/1 -> can_signal_process_wio_validity/5
  setvalid/1 -> can_signal_process_wio_validity/6
  ovrdflg/1 -> can_signal_process_wio_validity/7
  ovrdval/1 -> can_signal_process_wio_validity/8
  srvcflg/1 -> can_signal_process_wio_validity/9
  srvcval/1 -> can_signal_process_wio_validity/10
  Active_fault_flg/1 -> can_signal_process_wio_validity/3

== system inp/INP/TCM_signals/NowGearPosn ==
  signal_in : Inport
  signal_valid_in : Inport  Port=2
  msg_valid : Inport  Port=3
  Active_fault_flg : Inport  Port=4
  can_signal_process_with_validity : inp/can_signal_process_with_validity
  defval : Constant  Value=KINP_NowGearPosn_enum_defval
  invalid : Constant  Value=KINP_NowGearPosn_enum_invalid
  ovrdflg : Constant  Value=KINP_NowGearPosn_enum_ovrdflg
  ovrdval : Constant  Value=KINP_NowGearPosn_enum_ovrdval
  setvalid : Constant  Value=KINP_NowGearPosn_enum_setvalid
  srvcflg : Constant  Value=KINP_NowGearPosn_enum_srvcflg
  srvcval : Constant  Value=KINP_NowGearPosn_enum_srvcval
  signal_out : Outport
  signal_valid_out : Outport  Port=2
  raw_signal_valid_out : Outport  Port=3
  wires:
  Active_fault_flg/1 -> can_signal_process_with_validity/4
  msg_valid/1 -> can_signal_process_with_validity/3, can_signal_process_with_validity/5
  signal_valid_in/1 -> can_signal_process_with_validity/2
  signal_in/1 -> can_signal_process_with_validity/1
  can_signal_process_with_validity/2 -> signal_valid_out/1
  can_signal_process_with_validity/1 -> signal_out/1
  can_signal_process_with_validity/3 -> raw_signal_valid_out/1
  defval/1 -> can_signal_process_with_validity/6
  invalid/1 -> can_signal_process_with_validity/7
  setvalid/1 -> can_signal_process_with_validity/8
  ovrdflg/1 -> can_signal_process_with_validity/9
  ovrdval/1 -> can_signal_process_with_validity/10
  srvcflg/1 -> can_signal_process_with_validity/11
  srvcval/1 -> can_signal_process_with_validity/12

== system inp/INP/TCM_signals/ShifterPosn ==
  signal_in : Inport
  signal_valid_in : Inport  Port=2
  msg_valid : Inport  Port=3
  Active_fault_flg : Inport  Port=4
  can_signal_process_with_validity : inp/can_signal_process_with_validity
  defval : Constant  Value=KINP_ShifterPosn_enum_defval
  invalid : Constant  Value=KINP_ShifterPosn_enum_invalid
  ovrdflg : Constant  Value=KINP_ShifterPosn_enum_ovrdflg
  ovrdval : Constant  Value=KINP_ShifterPosn_enum_ovrdval
  setvalid : Constant  Value=KINP_ShifterPosn_enum_setvalid
  srvcflg : Constant  Value=KINP_ShifterPosn_enum_srvcflg
  srvcval : Constant  Value=KINP_ShifterPosn_enum_srvcval
  signal_out : Outport
  signal_valid_out : Outport  Port=2
  raw_signal_valid_out : Outport  Port=3
  wires:
  srvcval/1 -> can_signal_process_with_validity/12
  srvcflg/1 -> can_signal_process_with_validity/11
  ovrdval/1 -> can_signal_process_with_validity/10
  ovrdflg/1 -> can_signal_process_with_validity/9
  setvalid/1 -> can_signal_process_with_validity/8
  invalid/1 -> can_signal_process_with_validity/7
  defval/1 -> can_signal_process_with_validity/6
  can_signal_process_with_validity/3 -> raw_signal_valid_out/1
  can_signal_process_with_validity/1 -> signal_out/1
  can_signal_process_with_validity/2 -> signal_valid_out/1
  signal_in/1 -> can_signal_process_with_validity/1
  signal_valid_in/1 -> can_signal_process_with_validity/2
  msg_valid/1 -> can_signal_process_with_validity/5, can_signal_process_with_validity/3
  Active_fault_flg/1 -> can_signal_process_with_validity/4

== system inp/INP/TCM_signals/TCMFaultStatus ==
  signal_in : Inport
  msg_valid : Inport  Port=2
  Active_fault_flg : Inport  Port=3
  can_signal_process_wio_validity : inp/can_signal_process_wio_validity
  defval : Constant  Value=KINP_TCMFaultStatus_enum_defval
  invalid : Constant  Value=KINP_TCMFaultStatus_enum_invalid
  ovrdflg : Constant  Value=KINP_TCMFaultStatus_enum_ovrdflg
  ovrdval : Constant  Value=KINP_TCMFaultStatus_enum_ovrdval
  setvalid : Constant  Value=KINP_TCMFaultStatus_enum_setvalid
  srvcflg : Constant  Value=KINP_TCMFaultStatus_enum_srvcflg
  srvcval : Constant  Value=KINP_TCMFaultStatus_enum_srvcval
  signal_out : Outport
  signal_valid_out : Outport  Port=2
  wires:
  Active_fault_flg/1 -> can_signal_process_wio_validity/3
  srvcval/1 -> can_signal_process_wio_validity/10
  srvcflg/1 -> can_signal_process_wio_validity/9
  ovrdval/1 -> can_signal_process_wio_validity/8
  ovrdflg/1 -> can_signal_process_wio_validity/7
  setvalid/1 -> can_signal_process_wio_validity/6
  invalid/1 -> can_signal_process_wio_validity/5
  defval/1 -> can_signal_process_wio_validity/4
  msg_valid/1 -> can_signal_process_wio_validity/2
  signal_in/1 -> can_signal_process_wio_validity/1
  can_signal_process_wio_validity/2 -> signal_valid_out/1
  can_signal_process_wio_validity/1 -> signal_out/1

== system inp/INP/TCM_signals/TMTurbineSpd ==
  signal_in : Inport
  signal_valid_in : Inport  Port=2
  msg_valid : Inport  Port=3
  Active_fault_flg : Inport  Port=4
  can_signal_process_with_validity : inp/can_signal_process_with_validity
  conv_gain_offset : Subsystem
  defval : Constant  Value=KINP_TMTurbineSpd_rpm_defval
  invalid : Constant  Value=KINP_TMTurbineSpd_rpm_invalid
  min_max_limit : Subsystem
  ovrdflg : Constant  Value=KINP_TMTurbineSpd_rpm_ovrdflg
  ovrdval : Constant  Value=KINP_TMTurbineSpd_rpm_ovrdval
  setvalid : Constant  Value=KINP_TMTurbineSpd_rpm_setvalid
  srvcflg : Constant  Value=KINP_TMTurbineSpd_rpm_srvcflg
  srvcval : Constant  Value=KINP_TMTurbineSpd_rpm_srvcval
  signal_out : Outport
  signal_valid_out : Outport  Port=2
  raw_signal_valid_out : Outport  Port=3
  wires:
  srvcval/1 -> can_signal_process_with_validity/12
  srvcflg/1 -> can_signal_process_with_validity/11
  ovrdval/1 -> can_signal_process_with_validity/10
  ovrdflg/1 -> can_signal_process_with_validity/9
  setvalid/1 -> can_signal_process_with_validity/8
  invalid/1 -> can_signal_process_with_validity/7
  defval/1 -> can_signal_process_with_validity/6
  can_signal_process_with_validity/3 -> raw_signal_valid_out/1
  Active_fault_flg/1 -> can_signal_process_with_validity/4
  min_max_limit/1(out) -> signal_out/1
  conv_gain_offset/1(out) -> can_signal_process_with_validity/1
  msg_valid/1 -> can_signal_process_with_validity/5, can_signal_process_with_validity/3
  signal_valid_in/1 -> can_signal_process_with_validity/2
  signal_in/1 -> conv_gain_offset/1(in)
  can_signal_process_with_validity/2 -> signal_valid_out/1
  can_signal_process_with_validity/1 -> min_max_limit/1(in)

== system inp/INP/VCU_signals/ACCOnVCU ==
  signal_in : Inport
  msg_valid : Inport  Port=2
  Active_fault_flg : Inport  Port=3
  can_signal_process_wio_validity : inp/can_signal_process_wio_validity
  defval : Constant  Value=KINP_ACCOnVCU_flg_defval
  invalid : Constant  Value=KINP_ACCOnVCU_flg_invalid
  ovrdflg : Constant  Value=KINP_ACCOnVCU_flg_ovrdflg
  ovrdval : Constant  Value=KINP_ACCOnVCU_flg_ovrdval
  setvalid : Constant  Value=KINP_ACCOnVCU_flg_setvalid
  srvcflg : Constant  Value=KINP_ACCOnVCU_flg_srvcflg
  srvcval : Constant  Value=KINP_ACCOnVCU_flg_srvcval
  signal_out : Outport
  signal_valid_out : Outport  Port=2
  wires:
  can_signal_process_wio_validity/1 -> signal_out/1
  can_signal_process_wio_validity/2 -> signal_valid_out/1
  signal_in/1 -> can_signal_process_wio_validity/1
  msg_valid/1 -> can_signal_process_wio_validity/2
  defval/1 -> can_signal_process_wio_validity/4
  invalid/1 -> can_signal_process_wio_validity/5
  setvalid/1 -> can_signal_process_wio_validity/6
  ovrdflg/1 -> can_signal_process_wio_validity/7
  ovrdval/1 -> can_signal_process_wio_validity/8
  srvcflg/1 -> can_signal_process_wio_validity/9
  srvcval/1 -> can_signal_process_wio_validity/10
  Active_fault_flg/1 -> can_signal_process_wio_validity/3

== system inp/INP/VCU_signals/ACCResSpdIncVCU ==
  signal_in : Inport
  msg_valid : Inport  Port=2
  Active_fault_flg : Inport  Port=3
  can_signal_process_wio_validity : inp/can_signal_process_wio_validity
  defval : Constant  Value=KINP_ACCResSpdIncVCU_flg_defval
  invalid : Constant  Value=KINP_ACCResSpdIncVCU_flg_invalid
  ovrdflg : Constant  Value=KINP_ACCResSpdIncVCU_flg_ovrdflg
  ovrdval : Constant  Value=KINP_ACCResSpdIncVCU_flg_ovrdval
  setvalid : Constant  Value=KINP_ACCResSpdIncVCU_flg_setvalid
  srvcflg : Constant  Value=KINP_ACCResSpdIncVCU_flg_srvcflg
  srvcval : Constant  Value=KINP_ACCResSpdIncVCU_flg_srvcval
  signal_out : Outport
  signal_valid_out : Outport  Port=2
  wires:
  Active_fault_flg/1 -> can_signal_process_wio_validity/3
  srvcval/1 -> can_signal_process_wio_validity/10
  srvcflg/1 -> can_signal_process_wio_validity/9
  ovrdval/1 -> can_signal_process_wio_validity/8
  ovrdflg/1 -> can_signal_process_wio_validity/7
  setvalid/1 -> can_signal_process_wio_validity/6
  invalid/1 -> can_signal_process_wio_validity/5
  defval/1 -> can_signal_process_wio_validity/4
  msg_valid/1 -> can_signal_process_wio_validity/2
  signal_in/1 -> can_signal_process_wio_validity/1
  can_signal_process_wio_validity/2 -> signal_valid_out/1
  can_signal_process_wio_validity/1 -> signal_out/1

== system inp/INP/VCU_signals/ACCSetSpdDecVCU ==
  signal_in : Inport
  msg_valid : Inport  Port=2
  Active_fault_flg : Inport  Port=3
  can_signal_process_wio_validity : inp/can_signal_process_wio_validity
  defval : Constant  Value=KINP_ACCSetSpdDecVCU_flg_defval
  invalid : Constant  Value=KINP_ACCSetSpdDecVCU_flg_invalid
  ovrdflg : Constant  Value=KINP_ACCSetSpdDecVCU_flg_ovrdflg
  ovrdval : Constant  Value=KINP_ACCSetSpdDecVCU_flg_ovrdval
  setvalid : Constant  Value=KINP_ACCSetSpdDecVCU_flg_setvalid
  srvcflg : Constant  Value=KINP_ACCSetSpdDecVCU_flg_srvcflg
  srvcval : Constant  Value=KINP_ACCSetSpdDecVCU_flg_srvcval
  signal_out : Outport
  signal_valid_out : Outport  Port=2
  wires:
  can_signal_process_wio_validity/1 -> signal_out/1
  can_signal_process_wio_validity/2 -> signal_valid_out/1
  signal_in/1 -> can_signal_process_wio_validity/1
  msg_valid/1 -> can_signal_process_wio_validity/2
  defval/1 -> can_signal_process_wio_validity/4
  invalid/1 -> can_signal_process_wio_validity/5
  setvalid/1 -> can_signal_process_wio_validity/6
  ovrdflg/1 -> can_signal_process_wio_validity/7
  ovrdval/1 -> can_signal_process_wio_validity/8
  srvcflg/1 -> can_signal_process_wio_validity/9
  srvcval/1 -> can_signal_process_wio_validity/10
  Active_fault_flg/1 -> can_signal_process_wio_validity/3

== system inp/INP/VCU_signals/ACCTimeGapDecVCU ==
  signal_in : Inport
  msg_valid : Inport  Port=2
  Active_fault_flg : Inport  Port=3
  can_signal_process_wio_validity : inp/can_signal_process_wio_validity
  defval : Constant  Value=KINP_ACCTimeGapDecVCU_flg_defval
  invalid : Constant  Value=KINP_ACCTimeGapDecVCU_flg_invalid
  ovrdflg : Constant  Value=KINP_ACCTimeGapDecVCU_flg_ovrdflg
  ovrdval : Constant  Value=KINP_ACCTimeGapDecVCU_flg_ovrdval
  setvalid : Constant  Value=KINP_ACCTimeGapDecVCU_flg_setvalid
  srvcflg : Constant  Value=KINP_ACCTimeGapDecVCU_flg_srvcflg
  srvcval : Constant  Value=KINP_ACCTimeGapDecVCU_flg_srvcval
  signal_out : Outport
  signal_valid_out : Outport  Port=2
  wires:
  can_signal_process_wio_validity/1 -> signal_out/1
  can_signal_process_wio_validity/2 -> signal_valid_out/1
  signal_in/1 -> can_signal_process_wio_validity/1
  msg_valid/1 -> can_signal_process_wio_validity/2
  defval/1 -> can_signal_process_wio_validity/4
  invalid/1 -> can_signal_process_wio_validity/5
  setvalid/1 -> can_signal_process_wio_validity/6
  ovrdflg/1 -> can_signal_process_wio_validity/7
  ovrdval/1 -> can_signal_process_wio_validity/8
  srvcflg/1 -> can_signal_process_wio_validity/9
  srvcval/1 -> can_signal_process_wio_validity/10
  Active_fault_flg/1 -> can_signal_process_wio_validity/3

== system inp/INP/VCU_signals/ACCTimeGapIncVCU ==
  signal_in : Inport
  msg_valid : Inport  Port=2
  Active_fault_flg : Inport  Port=3
  can_signal_process_wio_validity : inp/can_signal_process_wio_validity
  defval : Constant  Value=KINP_ACCTimeGapIncVCU_flg_defval
  invalid : Constant  Value=KINP_ACCTimeGapIncVCU_flg_invalid
  ovrdflg : Constant  Value=KINP_ACCTimeGapIncVCU_flg_ovrdflg
  ovrdval : Constant  Value=KINP_ACCTimeGapIncVCU_flg_ovrdval
  setvalid : Constant  Value=KINP_ACCTimeGapIncVCU_flg_setvalid
  srvcflg : Constant  Value=KINP_ACCTimeGapIncVCU_flg_srvcflg
  srvcval : Constant  Value=KINP_ACCTimeGapIncVCU_flg_srvcval
  signal_out : Outport
  signal_valid_out : Outport  Port=2
  wires:
  Active_fault_flg/1 -> can_signal_process_wio_validity/3
  srvcval/1 -> can_signal_process_wio_validity/10
  srvcflg/1 -> can_signal_process_wio_validity/9
  ovrdval/1 -> can_signal_process_wio_validity/8
  ovrdflg/1 -> can_signal_process_wio_validity/7
  setvalid/1 -> can_signal_process_wio_validity/6
  invalid/1 -> can_signal_process_wio_validity/5
  defval/1 -> can_signal_process_wio_validity/4
  msg_valid/1 -> can_signal_process_wio_validity/2
  signal_in/1 -> can_signal_process_wio_validity/1
  can_signal_process_wio_validity/2 -> signal_valid_out/1
  can_signal_process_wio_validity/1 -> signal_out/1

== system inp/INP/VCU_signals/AckCurBrakDecReq ==
  signal_in : Inport
  signal_valid_in : Inport  Port=2
  msg_valid : Inport  Port=3
  Active_fault_flg : Inport  Port=4
  can_signal_process_with_validity : inp/can_signal_process_with_validity
  conv_gain_offset : Subsystem
  defval : Constant  Value=KINP_AckCurBrakDecReq_mps2_defval
  invalid : Constant  Value=KINP_AckCurBrakDecReq_mps2_invalid
  min_max_limit : Subsystem
  ovrdflg : Constant  Value=KINP_AckCurBrakDecReq_mps2_ovrdflg
  ovrdval : Constant  Value=KINP_AckCurBrakDecReq_mps2_ovrdval
  setvalid : Constant  Value=KINP_AckCurBrakDecReq_mps2_setvalid
  srvcflg : Constant  Value=KINP_AckCurBrakDecReq_mps2_srvcflg
  srvcval : Constant  Value=KINP_AckCurBrakDecReq_mps2_srvcval
  signal_out : Outport
  signal_valid_out : Outport  Port=2
  raw_signal_valid_out : Outport  Port=3
  wires:
  can_signal_process_with_validity/3 -> raw_signal_valid_out/1
  Active_fault_flg/1 -> can_signal_process_with_validity/4
  srvcval/1 -> can_signal_process_with_validity/12
  srvcflg/1 -> can_signal_process_with_validity/11
  ovrdval/1 -> can_signal_process_with_validity/10
  ovrdflg/1 -> can_signal_process_with_validity/9
  setvalid/1 -> can_signal_process_with_validity/8
  min_max_limit/1(out) -> signal_out/1
  conv_gain_offset/1(out) -> can_signal_process_with_validity/1
  invalid/1 -> can_signal_process_with_validity/7
  defval/1 -> can_signal_process_with_validity/6
  msg_valid/1 -> can_signal_process_with_validity/5, can_signal_process_with_validity/3
  signal_valid_in/1 -> can_signal_process_with_validity/2
  signal_in/1 -> conv_gain_offset/1(in)
  can_signal_process_with_validity/2 -> signal_valid_out/1
  can_signal_process_with_validity/1 -> min_max_limit/1(in)

== system inp/INP/VCU_signals/AckCurBrakDecReqApplable ==
  signal_in : Inport
  msg_valid : Inport  Port=2
  Active_fault_flg : Inport  Port=3
  can_signal_process_wio_validity : inp/can_signal_process_wio_validity
  defval : Constant  Value=KINP_AckCurBrakDecReqApplable_flg_defval
  invalid : Constant  Value=KINP_AckCurBrakDecReqApplable_flg_invalid
  ovrdflg : Constant  Value=KINP_AckCurBrakDecReqApplable_flg_ovrdflg
  ovrdval : Constant  Value=KINP_AckCurBrakDecReqApplable_flg_ovrdval
  setvalid : Constant  Value=KINP_AckCurBrakDecReqApplable_flg_setvalid
  srvcflg : Constant  Value=KINP_AckCurBrakDecReqApplable_flg_srvcflg
  srvcval : Constant  Value=KINP_AckCurBrakDecReqApplable_flg_srvcval
  signal_out : Outport
  signal_valid_out : Outport  Port=2
  wires:
  Active_fault_flg/1 -> can_signal_process_wio_validity/3
  srvcval/1 -> can_signal_process_wio_validity/10
  srvcflg/1 -> can_signal_process_wio_validity/9
  ovrdval/1 -> can_signal_process_wio_validity/8
  ovrdflg/1 -> can_signal_process_wio_validity/7
  setvalid/1 -> can_signal_process_wio_validity/6
  invalid/1 -> can_signal_process_wio_validity/5
  defval/1 -> can_signal_process_wio_validity/4
  msg_valid/1 -> can_signal_process_wio_validity/2
  signal_in/1 -> can_signal_process_wio_validity/1
  can_signal_process_wio_validity/2 -> signal_valid_out/1
  can_signal_process_wio_validity/1 -> signal_out/1

== system inp/INP/VCU_signals/AckCurPTTqReq ==
  signal_in : Inport
  signal_valid_in : Inport  Port=2
  msg_valid : Inport  Port=3
  Active_fault_flg : Inport  Port=4
  can_signal_process_with_validity : inp/can_signal_process_with_validity
  conv_gain_offset : Subsystem
  defval : Constant  Value=KINP_AckCurPTTqReq_Nm_defval
  invalid : Constant  Value=KINP_AckCurPTTqReq_Nm_invalid
  min_max_limit : Subsystem
  ovrdflg : Constant  Value=KINP_AckCurPTTqReq_Nm_ovrdflg
  ovrdval : Constant  Value=KINP_AckCurPTTqReq_Nm_ovrdval
  setvalid : Constant  Value=KINP_AckCurPTTqReq_Nm_setvalid
  srvcflg : Constant  Value=KINP_AckCurPTTqReq_Nm_srvcflg
  srvcval : Constant  Value=KINP_AckCurPTTqReq_Nm_srvcval
  signal_out : Outport
  signal_valid_out : Outport  Port=2
  raw_signal_valid_out : Outport  Port=3
  wires:
  can_signal_process_with_validity/1 -> min_max_limit/1(in)
  can_signal_process_with_validity/2 -> signal_valid_out/1
  signal_in/1 -> conv_gain_offset/1(in)
  signal_valid_in/1 -> can_signal_process_with_validity/2
  msg_valid/1 -> can_signal_process_with_validity/3, can_signal_process_with_validity/5
  defval/1 -> can_signal_process_with_validity/6
  invalid/1 -> can_signal_process_with_validity/7
  conv_gain_offset/1(out) -> can_signal_process_with_validity/1
  min_max_limit/1(out) -> signal_out/1
  setvalid/1 -> can_signal_process_with_validity/8
  ovrdflg/1 -> can_signal_process_with_validity/9
  ovrdval/1 -> can_signal_process_with_validity/10
  srvcflg/1 -> can_signal_process_with_validity/11
  srvcval/1 -> can_signal_process_with_validity/12
  Active_fault_flg/1 -> can_signal_process_with_validity/4
  can_signal_process_with_validity/3 -> raw_signal_valid_out/1
